simulink library "inp"  (Simulink 8.2)
settings: Solver=ode45  SolverMode=SingleTasking  StartTime=0.0  StopTime=10.0  RelTol=1e-3
undefined symbols (not defined in the file or in its folder): C_TICK_TIME_S, ENUM_FAULTSTATE_FAIL, ENUM_FAULTSTATE_INDETERMINATE, ENUM_FAULTSTATE_PASS, ENUM_KEYSWITCH_ON, FALSE, KINP_ABS1CANCommuOk_flg_ovrdflg, KINP_ABS1CANCommuOk_flg_ovrdval, KINP_ABS1CANPeriodTime_s, KINP_ABS1CANRolCntOk_flg_ovrdflg, KINP_ABS1CANRolCntOk_flg_ovrdval, KINP_ABS2CANChkSumOk_flg_ovrdflg, KINP_ABS2CANChkSumOk_flg_ovrdval, KINP_ABS2CANCommuOk_flg_ovrdflg, KINP_ABS2CANCommuOk_flg_ovrdval, KINP_ABS2CANPeriodTime_s, KINP_ABS2CANRolCntOk_flg_ovrdflg, KINP_ABS2CANRolCntOk_flg_ovrdval, KINP_ABS3CANCommuOk_flg_ovrdflg, KINP_ABS3CANCommuOk_flg_ovrdval, KINP_ABS4CANCommuOk_flg_ovrdflg, KINP_ABS4CANCommuOk_flg_ovrdval, KINP_ABSActive_flg_defval, KINP_ABSActive_flg_invalid, KINP_ABSActive_flg_ovrdflg, KINP_ABSActive_flg_ovrdval, KINP_ABSActive_flg_setvalid, KINP_ABSActive_flg_srvcflg, KINP_ABSActive_flg_srvcval, KINP_ABSCANAlive_flg_ovrdflg, KINP_ABSCANAlive_flg_ovrdval, KINP_ABSCANCommuOk_flg_ovrdflg, KINP_ABSCANCommuOk_flg_ovrdval, KINP_ABSCANValidOk_flg_ovrdflg, KINP_ABSCANValidOk_flg_ovrdval, KINP_ABSFault_flg_defval, KINP_ABSFault_flg_invalid, KINP_ABSFault_flg_ovrdflg, KINP_ABSFault_flg_ovrdval, KINP_ABSFault_flg_setvalid, KINP_ABSFault_flg_srvcflg, KINP_ABSFault_flg_srvcval, KINP_AC1CANCommuOk_flg_ovrdflg, KINP_AC1CANCommuOk_flg_ovrdval, KINP_ACCANAlive_flg_ovrdflg, KINP_ACCANAlive_flg_ovrdval, KINP_ACCANCommuOk_flg_ovrdflg, KINP_ACCANCommuOk_flg_ovrdval, KINP_ACCANValidOk_flg_ovrdflg, KINP_ACCANValidOk_flg_ovrdval, KINP_ACCCancelH_flg_defval, KINP_ACCCancelH_flg_invalid, KINP_ACCCancelH_flg_ovrdflg, KINP_ACCCancelH_flg_ovrdval, KINP_ACCCancelH_flg_setvalid, KINP_ACCCancelH_flg_srvcflg, KINP_ACCCancelH_flg_srvcval, KINP_AccelPedalPosn_pct_defval, KINP_AccelPedalPosn_pct_gain, KINP_AccelPedalPosn_pct_invalid ... (+1866)
libraries: inp x192, hcu_lib x34, simulink x33
(part 9 of 21)

== system inp/INP/ECM_signals/EngineIndicatedTorque ==
  signal_in : Inport
  signal_valid_in : Inport  Port=2
  msg_valid : Inport  Port=3
  Active_fault_flg : Inport  Port=4
  can_signal_process_with_validity : inp/can_signal_process_with_validity
  conv_gain_offset : Subsystem
  defval : Constant  Value=KINP_EngTqIndicated_Nm_defval
  invalid : Constant  Value=KINP_EngTqIndicated_Nm_invalid
  min_max_limit : Subsystem
  ovrdflg : Constant  Value=KINP_EngTqIndicated_Nm_ovrdflg
  ovrdval : Constant  Value=KINP_EngTqIndicated_Nm_ovrdval
  setvalid : Constant  Value=KINP_EngTqIndicated_Nm_setvalid
  srvcflg : Constant  Value=KINP_EngTqIndicated_Nm_srvcflg
  srvcval : Constant  Value=KINP_EngTqIndicated_Nm_srvcval
  signal_out : Outport
  signal_valid_out : Outport  Port=2
  raw_signal_valid_out : Outport  Port=3
  wires:
  can_signal_process_with_validity/1 -> min_max_limit/1(in)
  can_signal_process_with_validity/2 -> signal_valid_out/1
  signal_in/1 -> conv_gain_offset/1(in)
  signal_valid_in/1 -> can_signal_process_with_validity/2
  msg_valid/1 -> can_signal_process_with_validity/3, can_signal_process_with_validity/5
  conv_gain_offset/1(out) -> can_signal_process_with_validity/1
  min_max_limit/1(out) -> signal_out/1
  Active_fault_flg/1 -> can_signal_process_with_validity/4
  can_signal_process_with_validity/3 -> raw_signal_valid_out/1
  defval/1 -> can_signal_process_with_validity/6
  invalid/1 -> can_signal_process_with_validity/7
  setvalid/1 -> can_signal_process_with_validity/8
  ovrdflg/1 -> can_signal_process_with_validity/9
  ovrdval/1 -> can_signal_process_with_validity/10
  srvcflg/1 -> can_signal_process_with_validity/11
  srvcval/1 -> can_signal_process_with_validity/12

== system inp/INP/ECM_signals/EngineIndicatedTorqueCorrected ==
  signal_in : Inport
  signal_valid_in : Inport  Port=2
  msg_valid : Inport  Port=3
  Active_fault_flg : Inport  Port=4
  can_signal_process_with_validity : inp/can_signal_process_with_validity
  conv_gain_offset : Subsystem
  defval : Constant  Value=KINP_EngTqIndicatedCOR_Nm_defval
  invalid : Constant  Value=KINP_EngTqIndicatedCOR_Nm_invalid
  min_max_limit : Subsystem
  ovrdflg : Constant  Value=KINP_EngTqIndicatedCOR_Nm_ovrdflg
  ovrdval : Constant  Value=KINP_EngTqIndicatedCOR_Nm_ovrdval
  setvalid : Constant  Value=KINP_EngTqIndicatedCOR_Nm_setvalid
  srvcflg : Constant  Value=KINP_EngTqIndicatedCOR_Nm_srvcflg
  srvcval : Constant  Value=KINP_EngTqIndicatedCOR_Nm_srvcval
  signal_out : Outport
  signal_valid_out : Outport  Port=2
  raw_signal_valid_out : Outport  Port=3
  wires:
  srvcval/1 -> can_signal_process_with_validity/12
  srvcflg/1 -> can_signal_process_with_validity/11
  ovrdval/1 -> can_signal_process_with_validity/10
  ovrdflg/1 -> can_signal_process_with_validity/9
  setvalid/1 -> can_signal_process_with_validity/8
  invalid/1 -> can_signal_process_with_validity/7
  defval/1 -> can_signal_process_with_validity/6
  can_signal_process_with_validity/3 -> raw_signal_valid_out/1
  Active_fault_flg/1 -> can_signal_process_with_validity/4
  min_max_limit/1(out) -> signal_out/1
  conv_gain_offset/1(out) -> can_signal_process_with_validity/1
  msg_valid/1 -> can_signal_process_with_validity/5, can_signal_process_with_validity/3
  signal_valid_in/1 -> can_signal_process_with_validity/2
  signal_in/1 -> conv_gain_offset/1(in)
  can_signal_process_with_validity/2 -> signal_valid_out/1
  can_signal_process_with_validity/1 -> min_max_limit/1(in)

== system inp/INP/ECM_signals/EngineSpeed ==
  signal_in : Inport
  signal_valid_in : Inport  Port=2
  msg_valid : Inport  Port=3
  Active_fault_flg : Inport  Port=4
  can_signal_process_with_validity : inp/can_signal_process_with_validity
  conv_gain_offset : Subsystem
  defval : Constant  Value=KINP_EngSpd_erpm_defval
  invalid : Constant  Value=KINP_EngSpd_erpm_invalid
  min_max_limit : Subsystem
  ovrdflg : Constant  Value=KINP_EngSpd_erpm_ovrdflg
  ovrdval : Constant  Value=KINP_EngSpd_erpm_ovrdval
  setvalid : Constant  Value=KINP_EngSpd_erpm_setvalid
  srvcflg : Constant  Value=KINP_EngSpd_erpm_srvcflg
  srvcval : Constant  Value=KINP_EngSpd_erpm_srvcval
  signal_out : Outport
  signal_valid_out : Outport  Port=2
  raw_signal_valid_out : Outport  Port=3
  wires:
  can_signal_process_with_validity/1 -> min_max_limit/1(in)
  can_signal_process_with_validity/2 -> signal_valid_out/1
  signal_in/1 -> conv_gain_offset/1(in)
  signal_valid_in/1 -> can_signal_process_with_validity/2
  msg_valid/1 -> can_signal_process_with_validity/3, can_signal_process_with_validity/5
  conv_gain_offset/1(out) -> can_signal_process_with_validity/1
  min_max_limit/1(out) -> signal_out/1
  Active_fault_flg/1 -> can_signal_process_with_validity/4
  can_signal_process_with_validity/3 -> raw_signal_valid_out/1
  defval/1 -> can_signal_process_with_validity/6
  invalid/1 -> can_signal_process_with_validity/7
  setvalid/1 -> can_signal_process_with_validity/8
  ovrdflg/1 -> can_signal_process_with_validity/9
  ovrdval/1 -> can_signal_process_with_validity/10
  srvcflg/1 -> can_signal_process_with_validity/11
  srvcval/1 -> can_signal_process_with_validity/12

== system inp/INP/ECM_signals/EngineTorqueMax ==
  signal_in : Inport
  signal_valid_in : Inport  Port=2
  msg_valid : Inport  Port=3
  Active_fault_flg : Inport  Port=4
  can_signal_process_with_validity : inp/can_signal_process_with_validity
  conv_gain_offset : Subsystem
  defval : Constant  Value=KINP_EngTqMax_Nm_defval
  invalid : Constant  Value=KINP_EngTqMax_Nm_invalid
  min_max_limit : Subsystem
  ovrdflg : Constant  Value=KINP_EngTqMax_Nm_ovrdflg
  ovrdval : Constant  Value=KINP_EngTqMax_Nm_ovrdval
  setvalid : Constant  Value=KINP_EngTqMax_Nm_setvalid
  srvcflg : Constant  Value=KINP_EngTqMax_Nm_srvcflg
  srvcval : Constant  Value=KINP_EngTqMax_Nm_srvcval
  signal_out : Outport
  signal_valid_out : Outport  Port=2
  raw_signal_valid_out : Outport  Port=3
  wires:
  can_signal_process_with_validity/1 -> min_max_limit/1(in)
  can_signal_process_with_validity/2 -> signal_valid_out/1
  signal_in/1 -> conv_gain_offset/1(in)
  signal_valid_in/1 -> can_signal_process_with_validity/2
  msg_valid/1 -> can_signal_process_with_validity/3, can_signal_process_with_validity/5
  conv_gain_offset/1(out) -> can_signal_process_with_validity/1
  min_max_limit/1(out) -> signal_out/1
  Active_fault_flg/1 -> can_signal_process_with_validity/4
  can_signal_process_with_validity/3 -> raw_signal_valid_out/1
  defval/1 -> can_signal_process_with_validity/6
  invalid/1 -> can_signal_process_with_validity/7
  setvalid/1 -> can_signal_process_with_validity/8
  ovrdflg/1 -> can_signal_process_with_validity/9
  ovrdval/1 -> can_signal_process_with_validity/10
  srvcflg/1 -> can_signal_process_with_validity/11
  srvcval/1 -> can_signal_process_with_validity/12

== system inp/INP/ECM_signals/EngineTorqueMin ==
  signal_in : Inport
  signal_valid_in : Inport  Port=2
  msg_valid : Inport  Port=3
  Active_fault_flg : Inport  Port=4
  can_signal_process_with_validity : inp/can_signal_process_with_validity
  conv_gain_offset : Subsystem
  defval : Constant  Value=KINP_EngTqMin_Nm_defval
  invalid : Constant  Value=KINP_EngTqMin_Nm_invalid
  min_max_limit : Subsystem
  ovrdflg : Constant  Value=KINP_EngTqMin_Nm_ovrdflg
  ovrdval : Constant  Value=KINP_EngTqMin_Nm_ovrdval
  setvalid : Constant  Value=KINP_EngTqMin_Nm_setvalid
  srvcflg : Constant  Value=KINP_EngTqMin_Nm_srvcflg
  srvcval : Constant  Value=KINP_EngTqMin_Nm_srvcval
  signal_out : Outport
  signal_valid_out : Outport  Port=2
  raw_signal_valid_out : Outport  Port=3
  wires:
  srvcval/1 -> can_signal_process_with_validity/12
  srvcflg/1 -> can_signal_process_with_validity/11
  ovrdval/1 -> can_signal_process_with_validity/10
  ovrdflg/1 -> can_signal_process_with_validity/9
  setvalid/1 -> can_signal_process_with_validity/8
  invalid/1 -> can_signal_process_with_validity/7
  defval/1 -> can_signal_process_with_validity/6
  can_signal_process_with_validity/3 -> raw_signal_valid_out/1
  Active_fault_flg/1 -> can_signal_process_with_validity/4
  min_max_limit/1(out) -> signal_out/1
  conv_gain_offset/1(out) -> can_signal_process_with_validity/1
  msg_valid/1 -> can_signal_process_with_validity/5, can_signal_process_with_validity/3
  signal_valid_in/1 -> can_signal_process_with_validity/2
  signal_in/1 -> conv_gain_offset/1(in)
  can_signal_process_with_validity/2 -> signal_valid_out/1
  can_signal_process_with_validity/1 -> min_max_limit/1(in)

== system inp/INP/EPB_signals/ParkBrakeBindSta ==
  signal_in : Inport
  signal_valid_in : Inport  Port=2
  msg_valid : Inport  Port=3
  Active_fault_flg : Inport  Port=4
  can_signal_process_with_validity : inp/can_signal_process_with_validity
  defval : Constant  Value=KINP_ParkBrakeBindSta_enum_defval
  invalid : Constant  Value=KINP_ParkBrakeBindSta_enum_invalid
  ovrdflg : Constant  Value=KINP_ParkBrakeBindSta_enum_ovrdflg
  ovrdval : Constant  Value=KINP_ParkBrakeBindSta_enum_ovrdval
  setvalid : Constant  Value=KINP_ParkBrakeBindSta_enum_setvalid
  srvcflg : Constant  Value=KINP_ParkBrakeBindSta_enum_srvcflg
  srvcval : Constant  Value=KINP_ParkBrakeBindSta_enum_srvcval
  signal_out : Outport
  signal_valid_out : Outport  Port=2
  raw_signal_valid_out : Outport  Port=3
  wires:
  srvcval/1 -> can_signal_process_with_validity/12
  srvcflg/1 -> can_signal_process_with_validity/11
  ovrdval/1 -> can_signal_process_with_validity/10
  ovrdflg/1 -> can_signal_process_with_validity/9
  setvalid/1 -> can_signal_process_with_validity/8
  invalid/1 -> can_signal_process_with_validity/7
  defval/1 -> can_signal_process_with_validity/6
  can_signal_process_with_validity/3 -> raw_signal_valid_out/1
  can_signal_process_with_validity/1 -> signal_out/1
  can_signal_process_with_validity/2 -> signal_valid_out/1
  signal_in/1 -> can_signal_process_with_validity/1
  signal_valid_in/1 -> can_signal_process_with_validity/2
  msg_valid/1 -> can_signal_process_with_validity/5, can_signal_process_with_validity/3
  Active_fault_flg/1 -> can_signal_process_with_validity/4

== system inp/INP/EPM_signals/Cam_Left_Next_Lane ==
  BHAL_outputs : Inport
  CANvalid_bus : Inport  Port=2
  Bus Creator : BusCreator  Inputs=18
  CANVBusSel{1..9} (9 alike) : BusSelector  OutputSignals=VINP_CANMsgValidEPM_flg.VINP_CANMsgValidEPM1_flg
  Constant{1..9} (9 alike) : Constant  Value=TRUE
  Conv{1..4,7..9} (7 alike) : DataTypeConversion  OutDataTypeStr=single
  Conv5 : DataTypeConversion  OutDataTypeStr=uint8
  Conv6 : DataTypeConversion  OutDataTypeStr=uint8
  Gain{1..4} (4 alike) : Gain  Gain=-1
  HalBusSel1 : BusSelector  OutputSignals=VHAL_NextLaneC0L_m
  HalBusSel2 : BusSelector  OutputSignals=VHAL_NextLaneC1L_rad
  HalBusSel3 : BusSelector  OutputSignals=VHAL_NextLaneC2L_rat
  HalBusSel4 : BusSelector  OutputSignals=VHAL_NextLaneC3L_rat
  HalBusSel5 : BusSelector  OutputSignals=VHAL_NextLaneQualityL_enum
  HalBusSel6 : BusSelector  OutputSignals=VHAL_NextLaneTypeL_enum
  HalBusSel7 : BusSelector  OutputSignals=VHAL_NextLaneMrkWidL_m
  HalBusSel8 : BusSelector  OutputSignals=VHAL_NextViewRangeStartL_m
  HalBusSel9 : BusSelector  OutputSignals=VHAL_NextViewRangeEndL_m
  Term{1..9} (9 alike) : Terminator
  VINP_LeftNextLane_BUS_InHAL : Outport
  wires:
  Bus Creator/1 -> VINP_LeftNextLane_BUS_InHAL/1  "VINP_LeftNextLane_BUS_InHAL"
  CANvalid_bus/1 -> [CANvalid_bus]
  BHAL_outputs/1 -> [BHAL_outputs]
  Constant9/1 -> Bus Creator/18  "VINP_NextViewRangeEndLValid_flg_InHAL"
  Conv9/1 -> Bus Creator/17  "VINP_NextViewRangeEndL_m_InHAL"
  HalBusSel9/1 -> Conv9/1  "<VHAL_NextViewRangeEndL_m>"
  [BHAL_outputs] -> HalBusSel9/1
  Constant8/1 -> Bus Creator/16  "VINP_NextViewRangeStartLValid_flg_InHAL"
  Conv8/1 -> Bus Creator/15  "VINP_NextViewRangeStartL_m_InHAL"
  HalBusSel8/1 -> Conv8/1  "<VHAL_NextViewRangeStartL_m>"
  [BHAL_outputs] -> HalBusSel8/1
  Conv7/1 -> Bus Creator/13  "VINP_NextLaneMrkWidL_m_InHAL"
  Constant7/1 -> Bus Creator/14  "VINP_NextLaneMrkWidLValid_flg_InHAL"
  HalBusSel7/1 -> Conv7/1  "<VHAL_NextLaneMrkWidL_m>"
  [BHAL_outputs] -> HalBusSel7/1
  Constant6/1 -> Bus Creator/12  "VINP_NextLaneTypeLValid_flg_InHAL"
  Conv6/1 -> Bus Creator/11  "VINP_NextLaneTypeL_enum_InHAL"
  HalBusSel6/1 -> Conv6/1  "<VHAL_NextLaneTypeL_enum>"
  [BHAL_outputs] -> HalBusSel6/1
  Conv5/1 -> Bus Creator/9  "VINP_NextLaneQualityL_enum_InHAL"
  Constant5/1 -> Bus Creator/10  "VINP_NextLaneQualityLValid_flg_InHAL"
  [BHAL_outputs] -> HalBusSel5/1
  HalBusSel5/1 -> Conv5/1  "<VHAL_NextLaneQualityL_enum>"
  Conv4/1 -> Bus Creator/7  "VINP_NextLaneC3L_rat_InHAL"
  Constant4/1 -> Bus Creator/8  "VINP_NextLaneC3LValid_flg_InHAL"
  [BHAL_outputs] -> HalBusSel4/1
  HalBusSel4/1 -> Gain4/1  "<VHAL_NextLaneC3L_rat>"
  Conv3/1 -> Bus Creator/5  "VINP_NextLaneC2L_rat_InHAL"
  Constant3/1 -> Bus Creator/6  "VINP_NextLaneC2LValid_flg_InHAL"
  [BHAL_outputs] -> HalBusSel3/1
  HalBusSel3/1 -> Gain2/1  "<VHAL_NextLaneC2L_rat>"
  Conv2/1 -> Bus Creator/3  "VINP_NextLaneC1L_rad_InHAL"
  Constant2/1 -> Bus Creator/4  "VINP_NextLaneC1LValid_flg_InHAL"
  HalBusSel2/1 -> Gain1/1  "<VHAL_NextLaneC1L_rad>"
  [BHAL_outputs] -> HalBusSel2/1
  CANVBusSel1/1 -> Term1/1  "<VINP_CANMsgValidEPM1_flg>"
  Conv1/1 -> Bus Creator/1  "VINP_NextLaneC0L_m_InHAL"
  Constant1/1 -> Bus Creator/2  "VINP_NextLaneC0LValid_flg_InHAL"
  [CANvalid_bus] -> CANVBusSel1/1
  HalBusSel1/1 -> Gain3/1  "<VHAL_NextLaneC0L_m>"
  [BHAL_outputs] -> HalBusSel1/1
  CANVBusSel2/1 -> Term2/1  "<VINP_CANMsgValidEPM1_flg>"
  [CANvalid_bus] -> CANVBusSel2/1
  CANVBusSel3/1 -> Term3/1  "<VINP_CANMsgValidEPM1_flg>"
  [CANvalid_bus] -> CANVBusSel3/1
  CANVBusSel4/1 -> Term4/1  "<VINP_CANMsgValidEPM1_flg>"
  [CANvalid_bus] -> CANVBusSel4/1
  CANVBusSel5/1 -> Term5/1  "<VINP_CANMsgValidEPM1_flg>"
  [CANvalid_bus] -> CANVBusSel5/1
  CANVBusSel6/1 -> Term6/1  "<VINP_CANMsgValidEPM1_flg>"
  [CANvalid_bus] -> CANVBusSel6/1
  CANVBusSel7/1 -> Term7/1  "<VINP_CANMsgValidEPM1_flg>"
  [CANvalid_bus] -> CANVBusSel7/1
  CANVBusSel8/1 -> Term8/1  "<VINP_CANMsgValidEPM1_flg>"
  [CANvalid_bus] -> CANVBusSel8/1
  CANVBusSel9/1 -> Term9/1  "<VINP_CANMsgValidEPM1_flg>"
  [CANvalid_bus] -> CANVBusSel9/1
  Gain3/1 -> Conv1/1
  Gain1/1 -> Conv2/1
  Gain2/1 -> Conv3/1
  Gain4/1 -> Conv4/1

== system inp/INP/EPM_signals/Cam_Obstacle_1 ==
  BHAL_outputs : Inport
  CANvalid_bus : Inport  Port=2
  Bus Creator : BusCreator  Inputs=40
  CANVBusSel{1..10} (10 alike) : BusSelector  OutputSignals=VINP_CANMsgValidEPM_flg.VINP_CANMsgValidEPM6_flg
  CANVBusSel{11..15} (5 alike) : BusSelector  OutputSignals=VINP_CANMsgValidEPM_flg.VINP_CANMsgValidEPM7_flg
  CANVBusSel{16..20} (5 alike) : BusSelector  OutputSignals=VINP_CANMsgValidEPM_flg.VINP_CANMsgValidEPM8_flg
  Constant{1..20} (20 alike) : Constant  Value=TRUE
  Conv{1,5..9,13..14} (8 alike) : DataTypeConversion  OutDataTypeStr=uint8
  Conv10 : DataTypeConversion  OutDataTypeStr=boolean
  Conv{2..4,11..12,16..19} (9 alike) : DataTypeConversion  OutDataTypeStr=single
  Conv15 : DataTypeConversion  OutDataTypeStr=boolean
  Conv20 : DataTypeConversion  OutDataTypeStr=boolean
  HalBusSel1 : BusSelector  OutputSignals=VHAL_CamObs1ID_num
  HalBusSel10 : BusSelector  OutputSignals=VHAL_CamObs1BrakeLight_flg
  HalBusSel11 : BusSelector  OutputSignals=VHAL_CamObs1Length_m
  HalBusSel12 : BusSelector  OutputSignals=VHAL_CamObs1Width_m
  HalBusSel13 : BusSelector  OutputSignals=VHAL_CamObs1Age_cnt
  HalBusSel14 : BusSelector  OutputSignals=VHAL_CamObs1Lane_enum
  HalBusSel15 : BusSelector  OutputSignals=VHAL_CamObs1CIPV_flg
  HalBusSel16 : BusSelector  OutputSignals=VHAL_CamObs1VelY_mps
  HalBusSel17 : BusSelector  OutputSignals=VHAL_CamObs1AccX_mps2
  HalBusSel18 : BusSelector  OutputSignals=VHAL_CamObs1Ang_deg
  HalBusSel19 : BusSelector  OutputSignals=VHAL_CamObs1AngRate_degps
  HalBusSel2 : BusSelector  OutputSignals=VHAL_CamObs1PosX_m
  HalBusSel20 : BusSelector  OutputSignals=VHAL_CamObs1Replaced_flg
  HalBusSel3 : BusSelector  OutputSignals=VHAL_CamObs1PosY_m
  HalBusSel4 : BusSelector  OutputSignals=VHAL_CamObs1VelX_mps
  HalBusSel5 : BusSelector  OutputSignals=VHAL_CamObs1Type_enum
  HalBusSel6 : BusSelector  OutputSignals=VHAL_CamObs1Status_enum
  HalBusSel7 : BusSelector  OutputSignals=VHAL_CamObs1Valid_enum
  HalBusSel8 : BusSelector  OutputSignals=VHAL_CamObs1MoveInO_enum
  HalBusSel9 : BusSelector  OutputSignals=VHAL_CamObs1Blinker_enum
  Term{1..20} (20 alike) : Terminator
  Cam_Obstacle_1 : Outport
  wires:
  [BHAL_outputs] -> HalBusSel1/1
  HalBusSel1/1 -> Conv1/1  "<VHAL_CamObs1ID_num>"
  [CANvalid_bus] -> CANVBusSel1/1
  Constant1/1 -> Bus Creator/2  "VINP_CamObs1IDValid_flg_InHAL"
  Conv1/1 -> Bus Creator/1  "VINP_CamObs1ID_num_InHAL"
  CANVBusSel1/1 -> Term1/1  "<VINP_CANMsgValidEPM6_flg>"
  [CANvalid_bus] -> CANVBusSel2/1
  [BHAL_outputs] -> HalBusSel2/1
  HalBusSel2/1 -> Conv2/1  "<VHAL_CamObs1PosX_m>"
  Constant2/1 -> Bus Creator/4  "VINP_CamObs1PosXValid_flg_InHAL"
  Conv2/1 -> Bus Creator/3  "VINP_CamObs1PosX_m_InHAL"
  CANVBusSel2/1 -> Term2/1  "<VINP_CANMsgValidEPM6_flg>"
  HalBusSel3/1 -> Conv3/1  "<VHAL_CamObs1PosY_m>"
  [BHAL_outputs] -> HalBusSel3/1
  [CANvalid_bus] -> CANVBusSel3/1
  Constant3/1 -> Bus Creator/6  "VINP_CamObs1PosYValid_flg_InHAL"
  Conv3/1 -> Bus Creator/5  "VINP_CamObs1PosY_m_InHAL"
  CANVBusSel3/1 -> Term3/1  "<VINP_CANMsgValidEPM6_flg>"
  HalBusSel4/1 -> Conv4/1  "<VHAL_CamObs1VelX_mps>"
  [BHAL_outputs] -> HalBusSel4/1
  [CANvalid_bus] -> CANVBusSel4/1
  Constant4/1 -> Bus Creator/8  "VINP_CamObs1VelXValid_flg_InHAL"
  Conv4/1 -> Bus Creator/7  "VINP_CamObs1VelX_mps_InHAL"
  CANVBusSel4/1 -> Term4/1  "<VINP_CANMsgValidEPM6_flg>"
  HalBusSel5/1 -> Conv5/1  "<VHAL_CamObs1Type_enum>"
  [BHAL_outputs] -> HalBusSel5/1
  [CANvalid_bus] -> CANVBusSel5/1
  Constant5/1 -> Bus Creator/10  "VINP_CamObs1TypeValid_flg_InHAL"
  Conv5/1 -> Bus Creator/9  "VINP_CamObs1Type_enum_InHAL"
  CANVBusSel5/1 -> Term5/1  "<VINP_CANMsgValidEPM6_flg>"
  [BHAL_outputs] -> HalBusSel6/1
  HalBusSel6/1 -> Conv6/1  "<VHAL_CamObs1Status_enum>"
  Conv6/1 -> Bus Creator/11  "VINP_CamObs1Status_enum_InHAL"
  Constant6/1 -> Bus Creator/12  "VINP_CamObs1StatusValid_flg_InHAL"
  [CANvalid_bus] -> CANVBusSel6/1
  CANVBusSel6/1 -> Term6/1  "<VINP_CANMsgValidEPM6_flg>"
  [BHAL_outputs] -> HalBusSel7/1
  HalBusSel7/1 -> Conv7/1  "<VHAL_CamObs1Valid_enum>"
  [CANvalid_bus] -> CANVBusSel7/1
  Constant7/1 -> Bus Creator/14  "VINP_CamObs1ValidValid_flg_InHAL"
  Conv7/1 -> Bus Creator/13  "VINP_CamObs1Valid_enum_InHAL"
  CANVBusSel7/1 -> Term7/1  "<VINP_CANMsgValidEPM6_flg>"
  [BHAL_outputs] -> HalBusSel8/1
  HalBusSel8/1 -> Conv8/1  "<VHAL_CamObs1MoveInO_enum>"
  Conv8/1 -> Bus Creator/15  "VINP_CamObs1MoveInO_enum_InHAL"
  Constant8/1 -> Bus Creator/16  "VINP_CamObs1MoveInOValid_flg_InHAL"
  [CANvalid_bus] -> CANVBusSel8/1
  CANVBusSel8/1 -> Term8/1  "<VINP_CANMsgValidEPM6_flg>"
  [BHAL_outputs] -> HalBusSel9/1
  HalBusSel9/1 -> Conv9/1  "<VHAL_CamObs1Blinker_enum>"
  Conv9/1 -> Bus Creator/17  "VINP_CamObs1Blinker_enum_InHAL"
  Constant9/1 -> Bus Creator/18  "VINP_CamObs1BlinkerValid_flg_InHAL"
  [CANvalid_bus] -> CANVBusSel9/1
  CANVBusSel9/1 -> Term9/1  "<VINP_CANMsgValidEPM6_flg>"
  [BHAL_outputs] -> HalBusSel10/1
  HalBusSel10/1 -> Conv10/1  "<VHAL_CamObs1BrakeLight_flg>"
  Conv10/1 -> Bus Creator/19  "VINP_CamObs1BrakeLight_flg_InHAL"
  Constant10/1 -> Bus Creator/20  "VINP_CamObs1BrakeLightValid_flg_InHAL"
  [CANvalid_bus] -> CANVBusSel10/1
  CANVBusSel10/1 -> Term10/1  "<VINP_CANMsgValidEPM6_flg>"
  [BHAL_outputs] -> HalBusSel11/1
  [CANvalid_bus] -> CANVBusSel11/1
  HalBusSel11/1 -> Conv11/1  "<VHAL_CamObs1Length_m>"
  Constant11/1 -> Bus Creator/22  "VINP_CamObs1LengthValid_flg_InHAL"
  Conv11/1 -> Bus Creator/21  "VINP_CamObs1Length_m_InHAL"
  CANVBusSel11/1 -> Term11/1  "<VINP_CANMsgValidEPM7_flg>"
  [BHAL_outputs] -> HalBusSel12/1
  [CANvalid_bus] -> CANVBusSel12/1
  HalBusSel12/1 -> Conv12/1  "<VHAL_CamObs1Width_m>"
  Constant12/1 -> Bus Creator/24  "VINP_CamObs1WidthValid_flg_InHAL"
  Conv12/1 -> Bus Creator/23  "VINP_CamObs1Width_m_InHAL"
  CANVBusSel12/1 -> Term12/1  "<VINP_CANMsgValidEPM7_flg>"
  [BHAL_outputs] -> HalBusSel13/1
  [CANvalid_bus] -> CANVBusSel13/1
  HalBusSel13/1 -> Conv13/1  "<VHAL_CamObs1Age_cnt>"
  Constant13/1 -> Bus Creator/26  "VINP_CamObs1AgeValid_flg_InHAL"
  Conv13/1 -> Bus Creator/25  "VINP_CamObs1Age_cnt_InHAL"
  CANVBusSel13/1 -> Term13/1  "<VINP_CANMsgValidEPM7_flg>"
  HalBusSel14/1 -> Conv14/1  "<VHAL_CamObs1Lane_enum>"
  [BHAL_outputs] -> HalBusSel14/1
  [CANvalid_bus] -> CANVBusSel14/1
  Constant14/1 -> Bus Creator/28  "VINP_CamObs1LaneValid_flg_InHAL"
  Conv14/1 -> Bus Creator/27  "VINP_CamObs1Lane_enum_InHAL"
  CANVBusSel14/1 -> Term14/1  "<VINP_CANMsgValidEPM7_flg>"
  HalBusSel15/1 -> Conv15/1  "<VHAL_CamObs1CIPV_flg>"
  [BHAL_outputs] -> HalBusSel15/1
  [CANvalid_bus] -> CANVBusSel15/1
  Constant15/1 -> Bus Creator/30  "VINP_CamObs1CIPVValid_flg_InHAL"
  Conv15/1 -> Bus Creator/29  "VINP_CamObs1CIPV_flg_InHAL"
  CANVBusSel15/1 -> Term15/1  "<VINP_CANMsgValidEPM7_flg>"
  [BHAL_outputs] -> HalBusSel16/1
  [CANvalid_bus] -> CANVBusSel16/1
  HalBusSel16/1 -> Conv16/1  "<VHAL_CamObs1VelY_mps>"
  Constant16/1 -> Bus Creator/32  "VINP_CamObs1VelYValid_flg_InHAL"
  Conv16/1 -> Bus Creator/31  "VINP_CamObs1VelY_mps_InHAL"
  CANVBusSel16/1 -> Term16/1  "<VINP_CANMsgValidEPM8_flg>"
  [BHAL_outputs] -> HalBusSel17/1
  [CANvalid_bus] -> CANVBusSel17/1
  HalBusSel17/1 -> Conv17/1  "<VHAL_CamObs1AccX_mps2>"
  Constant17/1 -> Bus Creator/34  "VINP_CamObs1AccXValid_flg_InHAL"
  Conv17/1 -> Bus Creator/33  "VINP_CamObs1AccX_mps2_InHAL"
  CANVBusSel17/1 -> Term17/1  "<VINP_CANMsgValidEPM8_flg>"
  [BHAL_outputs] -> HalBusSel18/1
  [CANvalid_bus] -> CANVBusSel18/1
  HalBusSel18/1 -> Conv18/1  "<VHAL_CamObs1Ang_deg>"
  Constant18/1 -> Bus Creator/36  "VINP_CamObs1AngValid_flg_InHAL"
  Conv18/1 -> Bus Creator/35  "VINP_CamObs1Ang_deg_InHAL"
  CANVBusSel18/1 -> Term18/1  "<VINP_CANMsgValidEPM8_flg>"
  [BHAL_outputs] -> HalBusSel19/1
  HalBusSel19/1 -> Conv19/1  "<VHAL_CamObs1AngRate_degps>"
  Conv19/1 -> Bus Creator/37  "VINP_CamObs1AngRate_degps_InHAL"
  Constant19/1 -> Bus Creator/38  "VINP_CamObs1AngRateValid_flg_InHAL"
  [CANvalid_bus] -> CANVBusSel19/1
  CANVBusSel19/1 -> Term19/1  "<VINP_CANMsgValidEPM8_flg>"
  [BHAL_outputs] -> HalBusSel20/1
  HalBusSel20/1 -> Conv20/1  "<VHAL_CamObs1Replaced_flg>"
  Conv20/1 -> Bus Creator/39  "VINP_CamObs1Replaced_flg_InHAL"
  Constant20/1 -> Bus Creator/40  "VINP_CamObs1ReplacedValid_flg_InHAL"
  [CANvalid_bus] -> CANVBusSel20/1
  CANVBusSel20/1 -> Term20/1  "<VINP_CANMsgValidEPM8_flg>"
  BHAL_outputs/1 -> [BHAL_outputs]
  CANvalid_bus/1 -> [CANvalid_bus]
  Bus Creator/1 -> Cam_Obstacle_1/1  "VINP_Obstacle1_BUS_InHAL"

== system inp/INP/EPM_signals/Cam_Obstacle_10 ==
  BHAL_outputs : Inport
  CANvalid_bus : Inport  Port=2
  Bus Creator : BusCreator  Inputs=40
  CANVBusSel{181..190} (10 alike) : BusSelector  OutputSignals=VINP_CANMsgValidEPM_flg.VINP_CANMsgValidEPM33_flg
  CANVBusSel{191..195} (5 alike) : BusSelector  OutputSignals=VINP_CANMsgValidEPM_flg.VINP_CANMsgValidEPM34_flg
  CANVBusSel{196..200} (5 alike) : BusSelector  OutputSignals=VINP_CANMsgValidEPM_flg.VINP_CANMsgValidEPM35_flg
  Constant{181..200} (20 alike) : Constant  Value=TRUE
  Conv{181,185..189,193..194} (8 alike) : DataTypeConversion  OutDataTypeStr=uint8
  Conv{182..184,191..192,196..199} (9 alike) : DataTypeConversion  OutDataTypeStr=single
  Conv190 : DataTypeConversion  OutDataTypeStr=boolean
  Conv195 : DataTypeConversion  OutDataTypeStr=boolean
  Conv200 : DataTypeConversion  OutDataTypeStr=boolean
  HalBusSel181 : BusSelector  OutputSignals=VHAL_CamObs10ID_num
  HalBusSel182 : BusSelector  OutputSignals=VHAL_CamObs10PosX_m
  HalBusSel183 : BusSelector  OutputSignals=VHAL_CamObs10PosY_m
  HalBusSel184 : BusSelector  OutputSignals=VHAL_CamObs10VelX_mps
  HalBusSel185 : BusSelector  OutputSignals=VHAL_CamObs10Type_enum
  HalBusSel186 : BusSelector  OutputSignals=VHAL_CamObs10Status_enum
  HalBusSel187 : BusSelector  OutputSignals=VHAL_CamObs10Valid_enum
  HalBusSel188 : BusSelector  OutputSignals=VHAL_CamObs10MoveInO_enum
  HalBusSel189 : BusSelector  OutputSignals=VHAL_CamObs10Blinker_enum
  HalBusSel190 : BusSelector  OutputSignals=VHAL_CamObs10BrakeLight_flg
  HalBusSel191 : BusSelector  OutputSignals=VHAL_CamObs10Length_m
  HalBusSel192 : BusSelector  OutputSignals=VHAL_CamObs10Width_m
  HalBusSel193 : BusSelector  OutputSignals=VHAL_CamObs10Age_cnt
  HalBusSel194 : BusSelector  OutputSignals=VHAL_CamObs10Lane_enum
  HalBusSel195 : BusSelector  OutputSignals=VHAL_CamObs10CIPV_flg
  HalBusSel196 : BusSelector  OutputSignals=VHAL_CamObs10VelY_mps
  HalBusSel197 : BusSelector  OutputSignals=VHAL_CamObs10AccX_mps2
  HalBusSel198 : BusSelector  OutputSignals=VHAL_CamObs10Ang_deg
  HalBusSel199 : BusSelector  OutputSignals=VHAL_CamObs10AngRate_degps
  HalBusSel200 : BusSelector  OutputSignals=VHAL_CamObs10Replaced_flg
  Term{181..200} (20 alike) : Terminator
  Cam_Obstacle_10 : Outport
  wires:
  [BHAL_outputs] -> HalBusSel182/1
  [BHAL_outputs] -> HalBusSel190/1
  Constant181/1 -> Bus Creator/2  "VINP_CamObs10IDValid_flg_InHAL"
  CANVBusSel181/1 -> Term181/1  "<VINP_CANMsgValidEPM33_flg>"
  HalBusSel190/1 -> Conv190/1  "<VHAL_CamObs10BrakeLight_flg>"
  Conv190/1 -> Bus Creator/19  "VINP_CamObs10BrakeLight_flg_InHAL"
  [CANvalid_bus] -> CANVBusSel190/1
  CANVBusSel190/1 -> Term190/1  "<VINP_CANMsgValidEPM33_flg>"
  Conv182/1 -> Bus Creator/3  "VINP_CamObs10PosX_m_InHAL"
  [BHAL_outputs] -> HalBusSel184/1
  HalBusSel186/1 -> Conv186/1  "<VHAL_CamObs10Status_enum>"
  [CANvalid_bus] -> CANVBusSel188/1
  CANVBusSel188/1 -> Term188/1  "<VINP_CANMsgValidEPM33_flg>"
  Constant182/1 -> Bus Creator/4  "VINP_CamObs10PosXValid_flg_InHAL"
  [CANvalid_bus] -> CANVBusSel182/1
  Conv184/1 -> Bus Creator/7  "VINP_CamObs10VelX_mps_InHAL"
  HalBusSel184/1 -> Conv184/1  "<VHAL_CamObs10VelX_mps>"
  Constant184/1 -> Bus Creator/8  "VINP_CamObs10VelXValid_flg_InHAL"
  HalBusSel187/1 -> Conv187/1  "<VHAL_CamObs10Valid_enum>"
  HalBusSel189/1 -> Conv189/1  "<VHAL_CamObs10Blinker_enum>"
  Conv187/1 -> Bus Creator/13  "VINP_CamObs10Valid_enum_InHAL"
  [CANvalid_bus] -> CANVBusSel189/1
  HalBusSel185/1 -> Conv185/1  "<VHAL_CamObs10Type_enum>"
  CANVBusSel187/1 -> Term187/1  "<VINP_CANMsgValidEPM33_flg>"
  Constant187/1 -> Bus Creator/14  "VINP_CamObs10ValidValid_flg_InHAL"
  Conv189/1 -> Bus Creator/17  "VINP_CamObs10Blinker_enum_InHAL"
  Constant189/1 -> Bus Creator/18  "VINP_CamObs10BlinkerValid_flg_InHAL"
  Constant188/1 -> Bus Creator/16  "VINP_CamObs10MoveInOValid_flg_InHAL"
  [BHAL_outputs] -> HalBusSel188/1
  CANVBusSel189/1 -> Term189/1  "<VINP_CANMsgValidEPM33_flg>"
  CANVBusSel184/1 -> Term184/1  "<VINP_CANMsgValidEPM33_flg>"
  [BHAL_outputs] -> HalBusSel189/1
  [CANvalid_bus] -> CANVBusSel185/1
  [BHAL_outputs] -> HalBusSel187/1
  CANVBusSel186/1 -> Term186/1  "<VINP_CANMsgValidEPM33_flg>"
  [BHAL_outputs] -> HalBusSel186/1
  Conv185/1 -> Bus Creator/9  "VINP_CamObs10Type_enum_InHAL"
  Constant185/1 -> Bus Creator/10  "VINP_CamObs10TypeValid_flg_InHAL"
  [BHAL_outputs] -> HalBusSel185/1
  [CANvalid_bus] -> CANVBusSel184/1
  [BHAL_outputs] -> HalBusSel183/1
  Constant186/1 -> Bus Creator/12  "VINP_CamObs10StatusValid_flg_InHAL"
  CANVBusSel183/1 -> Term183/1  "<VINP_CANMsgValidEPM33_flg>"
  [CANvalid_bus] -> CANVBusSel183/1
  HalBusSel183/1 -> Conv183/1  "<VHAL_CamObs10PosY_m>"
  Conv186/1 -> Bus Creator/11  "VINP_CamObs10Status_enum_InHAL"
  CANVBusSel185/1 -> Term185/1  "<VINP_CANMsgValidEPM33_flg>"
  Conv183/1 -> Bus Creator/5  "VINP_CamObs10PosY_m_InHAL"
  [CANvalid_bus] -> CANVBusSel186/1
  Conv188/1 -> Bus Creator/15  "VINP_CamObs10MoveInO_enum_InHAL"
  [CANvalid_bus] -> CANVBusSel187/1
  CANVBusSel182/1 -> Term182/1  "<VINP_CANMsgValidEPM33_flg>"
  HalBusSel188/1 -> Conv188/1  "<VHAL_CamObs10MoveInO_enum>"
  Constant183/1 -> Bus Creator/6  "VINP_CamObs10PosYValid_flg_InHAL"
  [BHAL_outputs] -> HalBusSel181/1
  HalBusSel181/1 -> Conv181/1  "<VHAL_CamObs10ID_num>"
  [CANvalid_bus] -> CANVBusSel181/1
  Conv181/1 -> Bus Creator/1  "VINP_CamObs10ID_num_InHAL"
  HalBusSel182/1 -> Conv182/1  "<VHAL_CamObs10PosX_m>"
  Constant190/1 -> Bus Creator/20  "VINP_CamObs10BrakeLightValid_flg_InHAL"
  [BHAL_outputs] -> HalBusSel191/1
  HalBusSel191/1 -> Conv191/1  "<VHAL_CamObs10Length_m>"
  [CANvalid_bus] -> CANVBusSel191/1
  Conv191/1 -> Bus Creator/21  "VINP_CamObs10Length_m_InHAL"
  Constant191/1 -> Bus Creator/22  "VINP_CamObs10LengthValid_flg_InHAL"
  CANVBusSel191/1 -> Term191/1  "<VINP_CANMsgValidEPM34_flg>"
  [BHAL_outputs] -> HalBusSel192/1
  HalBusSel192/1 -> Conv192/1  "<VHAL_CamObs10Width_m>"
  [CANvalid_bus] -> CANVBusSel192/1
  Conv192/1 -> Bus Creator/23  "VINP_CamObs10Width_m_InHAL"
  Constant192/1 -> Bus Creator/24  "VINP_CamObs10WidthValid_flg_InHAL"
  CANVBusSel192/1 -> Term192/1  "<VINP_CANMsgValidEPM34_flg>"
  [BHAL_outputs] -> HalBusSel193/1
  HalBusSel193/1 -> Conv193/1  "<VHAL_CamObs10Age_cnt>"
  [CANvalid_bus] -> CANVBusSel193/1
  Conv193/1 -> Bus Creator/25  "VINP_CamObs10Age_cnt_InHAL"
  Constant193/1 -> Bus Creator/26  "VINP_CamObs10AgeValid_flg_InHAL"
  CANVBusSel193/1 -> Term193/1  "<VINP_CANMsgValidEPM34_flg>"
  [BHAL_outputs] -> HalBusSel194/1
  [CANvalid_bus] -> CANVBusSel194/1
  HalBusSel194/1 -> Conv194/1  "<VHAL_CamObs10Lane_enum>"
  Conv194/1 -> Bus Creator/27  "VINP_CamObs10Lane_enum_InHAL"
  Constant194/1 -> Bus Creator/28  "VINP_CamObs10LaneValid_flg_InHAL"
  CANVBusSel194/1 -> Term194/1  "<VINP_CANMsgValidEPM34_flg>"
  [BHAL_outputs] -> HalBusSel195/1
  HalBusSel195/1 -> Conv195/1  "<VHAL_CamObs10CIPV_flg>"
  [CANvalid_bus] -> CANVBusSel195/1
  Conv195/1 -> Bus Creator/29  "VINP_CamObs10CIPV_flg_InHAL"
  Constant195/1 -> Bus Creator/30  "VINP_CamObs10CIPVValid_flg_InHAL"
  CANVBusSel195/1 -> Term195/1  "<VINP_CANMsgValidEPM34_flg>"
  [BHAL_outputs] -> HalBusSel196/1
  HalBusSel196/1 -> Conv196/1  "<VHAL_CamObs10VelY_mps>"
  [CANvalid_bus] -> CANVBusSel196/1
  Conv196/1 -> Bus Creator/31  "VINP_CamObs10VelY_mps_InHAL"
  Constant196/1 -> Bus Creator/32  "VINP_CamObs10VelYValid_flg_InHAL"
  CANVBusSel196/1 -> Term196/1  "<VINP_CANMsgValidEPM35_flg>"
  [BHAL_outputs] -> HalBusSel197/1
  [CANvalid_bus] -> CANVBusSel197/1
  HalBusSel197/1 -> Conv197/1  "<VHAL_CamObs10AccX_mps2>"
  Conv197/1 -> Bus Creator/33  "VINP_CamObs10AccX_mps2_InHAL"
  Constant197/1 -> Bus Creator/34  "VINP_CamObs10AccXValid_flg_InHAL"
  CANVBusSel197/1 -> Term197/1  "<VINP_CANMsgValidEPM35_flg>"
  [BHAL_outputs] -> HalBusSel198/1
  HalBusSel198/1 -> Conv198/1  "<VHAL_CamObs10Ang_deg>"
  [CANvalid_bus] -> CANVBusSel198/1
  Conv198/1 -> Bus Creator/35  "VINP_CamObs10Ang_deg_InHAL"
  Constant198/1 -> Bus Creator/36  "VINP_CamObs10AngValid_flg_InHAL"
  CANVBusSel198/1 -> Term198/1  "<VINP_CANMsgValidEPM35_flg>"
  [BHAL_outputs] -> HalBusSel199/1
  Conv199/1 -> Bus Creator/37  "VINP_CamObs10AngRate_degps_InHAL"
  HalBusSel199/1 -> Conv199/1  "<VHAL_CamObs10AngRate_degps>"
  [CANvalid_bus] -> CANVBusSel199/1
  Constant199/1 -> Bus Creator/38  "VINP_CamObs10AngRateValid_flg_InHAL"
  CANVBusSel199/1 -> Term199/1  "<VINP_CANMsgValidEPM35_flg>"
  [BHAL_outputs] -> HalBusSel200/1
  Conv200/1 -> Bus Creator/39  "VINP_CamObs10Replaced_flg_InHAL"
  HalBusSel200/1 -> Conv200/1  "<VHAL_CamObs10Replaced_flg>"
  [CANvalid_bus] -> CANVBusSel200/1
  Constant200/1 -> Bus Creator/40  "VINP_CamObs10ReplacedValid_flg_InHAL"
  CANVBusSel200/1 -> Term200/1  "<VINP_CANMsgValidEPM35_flg>"
  BHAL_outputs/1 -> [BHAL_outputs]
  CANvalid_bus/1 -> [CANvalid_bus]
  Bus Creator/1 -> Cam_Obstacle_10/1  "VINP_Obstacle10_BUS_InHAL"

== system inp/INP/EPM_signals/Cam_Obstacle_2 ==
  BHAL_outputs : Inport
  CANvalid_bus : Inport  Port=2
  Bus Creator : BusCreator  Inputs=40
  CANVBusSel{21..30} (10 alike) : BusSelector  OutputSignals=VINP_CANMsgValidEPM_flg.VINP_CANMsgValidEPM9_flg
  CANVBusSel{31..35} (5 alike) : BusSelector  OutputSignals=VINP_CANMsgValidEPM_flg.VINP_CANMsgValidEPM10_flg
  CANVBusSel{36..40} (5 alike) : BusSelector  OutputSignals=VINP_CANMsgValidEPM_flg.VINP_CANMsgValidEPM11_flg
  Constant{21..40} (20 alike) : Constant  Value=TRUE
  Conv{21,25..29,33..34} (8 alike) : DataTypeConversion  OutDataTypeStr=uint8
  Conv{22..24,31..32,36..39} (9 alike) : DataTypeConversion  OutDataTypeStr=single
  Conv30 : DataTypeConversion  OutDataTypeStr=boolean
  Conv35 : DataTypeConversion  OutDataTypeStr=boolean
  Conv40 : DataTypeConversion  OutDataTypeStr=boolean
  HalBusSel21 : BusSelector  OutputSignals=VHAL_CamObs2ID_num
  HalBusSel22 : BusSelector  OutputSignals=VHAL_CamObs2PosX_m
  HalBusSel23 : BusSelector  OutputSignals=VHAL_CamObs2PosY_m
  HalBusSel24 : BusSelector  OutputSignals=VHAL_CamObs2VelX_mps
  HalBusSel25 : BusSelector  OutputSignals=VHAL_CamObs2Type_enum
  HalBusSel26 : BusSelector  OutputSignals=VHAL_CamObs2Status_enum
  HalBusSel27 : BusSelector  OutputSignals=VHAL_CamObs2Valid_enum
  HalBusSel28 : BusSelector  OutputSignals=VHAL_CamObs2MoveInO_enum
  HalBusSel29 : BusSelector  OutputSignals=VHAL_CamObs2Blinker_enum
  HalBusSel30 : BusSelector  OutputSignals=VHAL_CamObs2BrakeLight_flg
  HalBusSel31 : BusSelector  OutputSignals=VHAL_CamObs2Length_m
  HalBusSel32 : BusSelector  OutputSignals=VHAL_CamObs2Width_m
  HalBusSel33 : BusSelector  OutputSignals=VHAL_CamObs2Age_cnt
  HalBusSel34 : BusSelector  OutputSignals=VHAL_CamObs2Lane_enum
  HalBusSel35 : BusSelector  OutputSignals=VHAL_CamObs2CIPV_flg
  HalBusSel36 : BusSelector  OutputSignals=VHAL_CamObs2VelY_mps
  HalBusSel37 : BusSelector  OutputSignals=VHAL_CamObs2AccX_mps2
  HalBusSel38 : BusSelector  OutputSignals=VHAL_CamObs2Ang_deg
  HalBusSel39 : BusSelector  OutputSignals=VHAL_CamObs2AngRate_degps
  HalBusSel40 : BusSelector  OutputSignals=VHAL_CamObs2Replaced_flg
  Term{21..40} (20 alike) : Terminator
  Cam_Obstacle_2 : Outport
  wires:
  HalBusSel21/1 -> Conv21/1  "<VHAL_CamObs2ID_num>"
  [BHAL_outputs] -> HalBusSel21/1
  [CANvalid_bus] -> CANVBusSel21/1
  Constant21/1 -> Bus Creator/2  "VINP_CamObs2IDValid_flg_InHAL"
  Conv21/1 -> Bus Creator/1  "VINP_CamObs2ID_num_InHAL"
  CANVBusSel21/1 -> Term21/1  "<VINP_CANMsgValidEPM9_flg>"
  [CANvalid_bus] -> CANVBusSel22/1
  [BHAL_outputs] -> HalBusSel22/1
  HalBusSel22/1 -> Conv22/1  "<VHAL_CamObs2PosX_m>"
  Constant22/1 -> Bus Creator/4  "VINP_CamObs2PosXValid_flg_InHAL"
  Conv22/1 -> Bus Creator/3  "VINP_CamObs2PosX_m_InHAL"
  CANVBusSel22/1 -> Term22/1  "<VINP_CANMsgValidEPM9_flg>"
  HalBusSel23/1 -> Conv23/1  "<VHAL_CamObs2PosY_m>"
  [BHAL_outputs] -> HalBusSel23/1
  [CANvalid_bus] -> CANVBusSel23/1
  Constant23/1 -> Bus Creator/6  "VINP_CamObs2PosYValid_flg_InHAL"
  Conv23/1 -> Bus Creator/5  "VINP_CamObs2PosY_m_InHAL"
  CANVBusSel23/1 -> Term23/1  "<VINP_CANMsgValidEPM9_flg>"
  HalBusSel24/1 -> Conv24/1  "<VHAL_CamObs2VelX_mps>"
  [BHAL_outputs] -> HalBusSel24/1
  [CANvalid_bus] -> CANVBusSel24/1
  Constant24/1 -> Bus Creator/8  "VINP_CamObs2VelXValid_flg_InHAL"
  Conv24/1 -> Bus Creator/7  "VINP_CamObs2VelX_mps_InHAL"
  CANVBusSel24/1 -> Term24/1  "<VINP_CANMsgValidEPM9_flg>"
  HalBusSel25/1 -> Conv25/1  "<VHAL_CamObs2Type_enum>"
  [BHAL_outputs] -> HalBusSel25/1
  [CANvalid_bus] -> CANVBusSel25/1
  Constant25/1 -> Bus Creator/10  "VINP_CamObs2TypeValid_flg_InHAL"
  Conv25/1 -> Bus Creator/9  "VINP_CamObs2Type_enum_InHAL"
  CANVBusSel25/1 -> Term25/1  "<VINP_CANMsgValidEPM9_flg>"
  [BHAL_outputs] -> HalBusSel26/1
  HalBusSel26/1 -> Conv26/1  "<VHAL_CamObs2Status_enum>"
  Conv26/1 -> Bus Creator/11  "VINP_CamObs2Status_enum_InHAL"
  Constant26/1 -> Bus Creator/12  "VINP_CamObs2StatusValid_flg_InHAL"
  [CANvalid_bus] -> CANVBusSel26/1
  CANVBusSel26/1 -> Term26/1  "<VINP_CANMsgValidEPM9_flg>"
  [BHAL_outputs] -> HalBusSel27/1
  HalBusSel27/1 -> Conv27/1  "<VHAL_CamObs2Valid_enum>"
  [CANvalid_bus] -> CANVBusSel27/1
  Constant27/1 -> Bus Creator/14  "VINP_CamObs2ValidValid_flg_InHAL"
  Conv27/1 -> Bus Creator/13  "VINP_CamObs2Valid_enum_InHAL"
  CANVBusSel27/1 -> Term27/1  "<VINP_CANMsgValidEPM9_flg>"
  [BHAL_outputs] -> HalBusSel28/1
  HalBusSel28/1 -> Conv28/1  "<VHAL_CamObs2MoveInO_enum>"
  Conv28/1 -> Bus Creator/15  "VINP_CamObs2MoveInO_enum_InHAL"
  Constant28/1 -> Bus Creator/16  "VINP_CamObs2MoveInOValid_flg_InHAL"
  [CANvalid_bus] -> CANVBusSel28/1
  CANVBusSel28/1 -> Term28/1  "<VINP_CANMsgValidEPM9_flg>"
  [BHAL_outputs] -> HalBusSel29/1
  HalBusSel29/1 -> Conv29/1  "<VHAL_CamObs2Blinker_enum>"
  Conv29/1 -> Bus Creator/17  "VINP_CamObs2Blinker_enum_InHAL"
  Constant29/1 -> Bus Creator/18  "VINP_CamObs2BlinkerValid_flg_InHAL"
  [CANvalid_bus] -> CANVBusSel29/1
  CANVBusSel29/1 -> Term29/1  "<VINP_CANMsgValidEPM9_flg>"
  [BHAL_outputs] -> HalBusSel30/1
  HalBusSel30/1 -> Conv30/1  "<VHAL_CamObs2BrakeLight_flg>"
  Conv30/1 -> Bus Creator/19  "VINP_CamObs2BrakeLight_flg_InHAL"
  Constant30/1 -> Bus Creator/20  "VINP_CamObs2BrakeLightValid_flg_InHAL"
  [CANvalid_bus] -> CANVBusSel30/1
  CANVBusSel30/1 -> Term30/1  "<VINP_CANMsgValidEPM9_flg>"
  [BHAL_outputs] -> HalBusSel31/1
  [CANvalid_bus] -> CANVBusSel31/1
  HalBusSel31/1 -> Conv31/1  "<VHAL_CamObs2Length_m>"
  Constant31/1 -> Bus Creator/22  "VINP_CamObs2LengthValid_flg_InHAL"
  Conv31/1 -> Bus Creator/21  "VINP_CamObs2Length_m_InHAL"
  CANVBusSel31/1 -> Term31/1  "<VINP_CANMsgValidEPM10_flg>"
  [BHAL_outputs] -> HalBusSel32/1
  [CANvalid_bus] -> CANVBusSel32/1
  HalBusSel32/1 -> Conv32/1  "<VHAL_CamObs2Width_m>"
  Constant32/1 -> Bus Creator/24  "VINP_CamObs2WidthValid_flg_InHAL"
  Conv32/1 -> Bus Creator/23  "VINP_CamObs2Width_m_InHAL"
  CANVBusSel32/1 -> Term32/1  "<VINP_CANMsgValidEPM10_flg>"
  [BHAL_outputs] -> HalBusSel33/1
  [CANvalid_bus] -> CANVBusSel33/1
  HalBusSel33/1 -> Conv33/1  "<VHAL_CamObs2Age_cnt>"
  Constant33/1 -> Bus Creator/26  "VINP_CamObs2AgeValid_flg_InHAL"
  Conv33/1 -> Bus Creator/25  "VINP_CamObs2Age_cnt_InHAL"
  CANVBusSel33/1 -> Term33/1  "<VINP_CANMsgValidEPM10_flg>"
  HalBusSel34/1 -> Conv34/1  "<VHAL_CamObs2Lane_enum>"
  [BHAL_outputs] -> HalBusSel34/1
  [CANvalid_bus] -> CANVBusSel34/1
  Constant34/1 -> Bus Creator/28  "VINP_CamObs2LaneValid_flg_InHAL"
  Conv34/1 -> Bus Creator/27  "VINP_CamObs2Lane_enum_InHAL"
  CANVBusSel34/1 -> Term34/1  "<VINP_CANMsgValidEPM10_flg>"
  HalBusSel35/1 -> Conv35/1  "<VHAL_CamObs2CIPV_flg>"
  [BHAL_outputs] -> HalBusSel35/1
  [CANvalid_bus] -> CANVBusSel35/1
  Constant35/1 -> Bus Creator/30  "VINP_CamObs2CIPVValid_flg_InHAL"
  Conv35/1 -> Bus Creator/29  "VINP_CamObs2CIPV_flg_InHAL"
  CANVBusSel35/1 -> Term35/1  "<VINP_CANMsgValidEPM10_flg>"
  [BHAL_outputs] -> HalBusSel36/1
  [CANvalid_bus] -> CANVBusSel36/1
  HalBusSel36/1 -> Conv36/1  "<VHAL_CamObs2VelY_mps>"
  Constant36/1 -> Bus Creator/32  "VINP_CamObs2VelYValid_flg_InHAL"
  Conv36/1 -> Bus Creator/31  "VINP_CamObs2VelY_mps_InHAL"
  CANVBusSel36/1 -> Term36/1  "<VINP_CANMsgValidEPM11_flg>"
  [BHAL_outputs] -> HalBusSel37/1
  [CANvalid_bus] -> CANVBusSel37/1
  HalBusSel37/1 -> Conv37/1  "<VHAL_CamObs2AccX_mps2>"
  Constant37/1 -> Bus Creator/34  "VINP_CamObs2AccXValid_flg_InHAL"
  Conv37/1 -> Bus Creator/33  "VINP_CamObs2AccX_mps2_InHAL"
  CANVBusSel37/1 -> Term37/1  "<VINP_CANMsgValidEPM11_flg>"
  [BHAL_outputs] -> HalBusSel38/1
  [CANvalid_bus] -> CANVBusSel38/1
  HalBusSel38/1 -> Conv38/1  "<VHAL_CamObs2Ang_deg>"
  Constant38/1 -> Bus Creator/36  "VINP_CamObs2AngValid_flg_InHAL"
  Conv38/1 -> Bus Creator/35  "VINP_CamObs2Ang_deg_InHAL"
  CANVBusSel38/1 -> Term38/1  "<VINP_CANMsgValidEPM11_flg>"
  [BHAL_outputs] -> HalBusSel39/1
  HalBusSel39/1 -> Conv39/1  "<VHAL_CamObs2AngRate_degps>"
  Conv39/1 -> Bus Creator/37  "VINP_CamObs2AngRate_degps_InHAL"
  Constant39/1 -> Bus Creator/38  "VINP_CamObs2AngRateValid_flg_InHAL"
  [CANvalid_bus] -> CANVBusSel39/1
  CANVBusSel39/1 -> Term39/1  "<VINP_CANMsgValidEPM11_flg>"
  [BHAL_outputs] -> HalBusSel40/1
  HalBusSel40/1 -> Conv40/1  "<VHAL_CamObs2Replaced_flg>"
  Conv40/1 -> Bus Creator/39  "VINP_CamObs2Replaced_flg_InHAL"
  Constant40/1 -> Bus Creator/40  "VINP_CamObs2ReplacedValid_flg_InHAL"
  [CANvalid_bus] -> CANVBusSel40/1
  CANVBusSel40/1 -> Term40/1  "<VINP_CANMsgValidEPM11_flg>"
  BHAL_outputs/1 -> [BHAL_outputs]
  CANvalid_bus/1 -> [CANvalid_bus]
  Bus Creator/1 -> Cam_Obstacle_2/1  "VINP_Obstacle2_BUS_InHAL"
